simulink model "SunLoadCalcn"  (R2022b)
settings: Solver=FixedStepDiscrete  StartTime=0.0  StopTime=10.0  FixedStep=1  RelTol=1e-3
settings Model: Creator=blue.ge  LastModifiedBy=blue.ge
settings DataIOCC: SaveFormat=StructureWithTime
settings OptimizationCC: DefaultParameterBehavior=Inlined  EnhancedBackFolding=on  PassReuseOutputArgsAs=Individual arguments  ZeroExternalMemoryAtStartup=off  ZeroInternalMemoryAtStartup=off  OptimizeBlockOrder=speed  DifferentSizesBufferReuse=on  OptimizationCustomize=off  EfficientTunableParamExpr=on
settings DebuggingCC: ParameterTunabilityLossMsg=error
settings ModelReferenceCC: ModelReferenceNumInstancesAllowed=Single
settings SFSimCC: MATLABDynamicMemAlloc=off
settings RTWCC: Description=AUTOSAR  SystemTargetFile=autosar.tlc  GenCodeOnly=on  TemplateMakefile=ert_default_tmf
settings CodeAppCC: ForceParamTrailComments=on  IgnoreCustomStorageClasses=off  OperatorAnnotations=on  SimulinkDataObjDesc=on  SFDataObjDesc=on  InsertBlockDesc=on
settings STFCustomTargetCC: TargetLangStandard=C99 (ISO)  MultiwordLength=256  ParMdlRefBuildCompliant=on  CompOptLevelCompliant=on  IncludeMdlTerminateFcn=off  CombineOutputUpdateFcns=on  SuppressErrorStatus=on  ERTCustomFileBanners=on  SupportNonFinite=off  SupportComplex=off  SupportContinuousTime=off  SupportNonInlinedSFcns=off  RemoveResetFunc=on  MATLABClassNameForMDSCustomization=Simulink.SoftwareTarget.ERTCustomization  AutosarCompliant=on  UseToolchainInfoCompliant=on  LookupTableObjectStructAxisOrder=2,1,3,4,...  LUTObjectStructOrderEvenSpacing=Size,Table,Breakpoints  SystemTargetFile=autosar.tlc
settings ERTTargetCC: TargetLangStandard=C99 (ISO)  ConcurrentExecutionCompliant=off  IncludeMdlTerminateFcn=off  SuppressErrorStatus=on  SupportNonFinite=off  SupportComplex=off  ModelStepFunctionPrototypeControlCompliant=off  CPPClassGenCompliant=off  AutosarCompliant=on  LookupTableObjectStructAxisOrder=2,1,3,4,...  LUTObjectStructOrderEvenSpacing=Size,Table,Breakpoints  InlinedParameterPlacement=NonHierarchical  ERTCustomFileTemplate=example_file_process.tlc

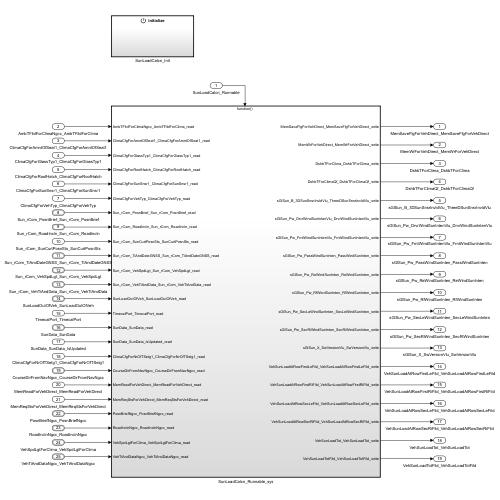
[thumbnail: Simulink's stored preview of the top-level diagram]
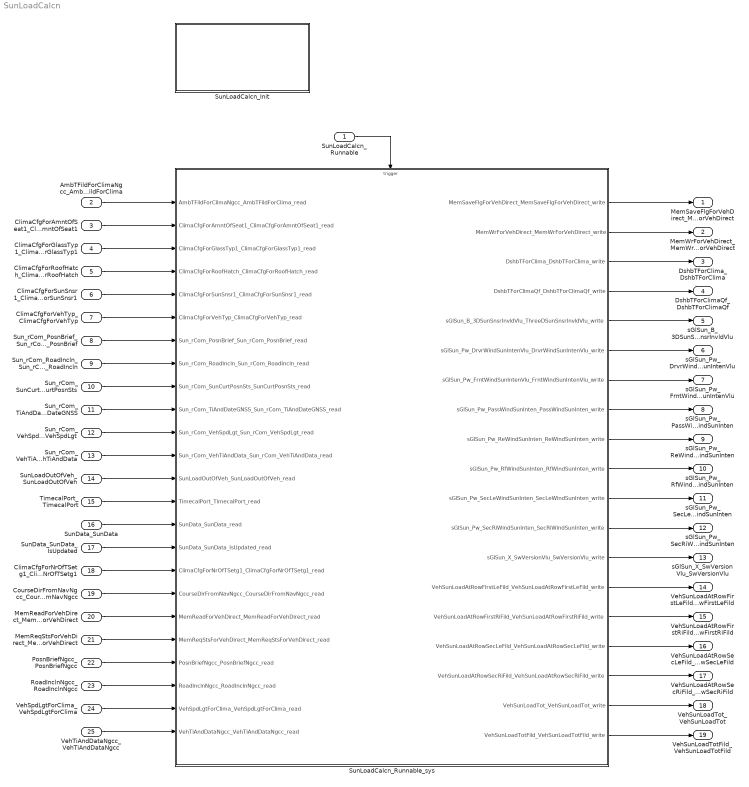
== system SunLoadCalcn ==
  SunLoadCalcn_Runnable : Inport  SampleTime=1  OutputFunctionCall=on
  AmbTFildForClimaNgcc_AmbTFildForClima : Inport  Port=2  OutDataTypeStr=T30  PortDimensions=1
  ClimaCfgForAmntOfSeat1_ClimaCfgForAmntOfSeat1 : Inport  Port=3  OutDataTypeStr=Enum: ClimaCfgForAmntOfSeat1  PortDimensions=1
  ClimaCfgForGlassTyp1_ClimaCfgForGlassTyp1 : Inport  Port=4  OutDataTypeStr=Enum: ClimaCfgForGlassTyp1  PortDimensions=1
  ClimaCfgForRoofHatch_ClimaCfgForRoofHatch : Inport  Port=5  OutDataTypeStr=Enum: CarCfgForRoofHatch  PortDimensions=1
  ClimaCfgForSunSnsr1_ClimaCfgForSunSnsr1 : Inport  Port=6  OutDataTypeStr=Enum: CarCfgForSunSnsr  PortDimensions=1
  ClimaCfgForVehTyp_ClimaCfgForVehTyp : Inport  Port=7  OutDataTypeStr=Enum: CarCfgForVehTyp3  PortDimensions=1
  Sun_rCom_PosnBrief_Sun_rCom_PosnBrief : Inport  Port=8  OutDataTypeStr=Bus: Posn  PortDimensions=1  BusOutputAsStruct=on
  Sun_rCom_RoadIncln_Sun_rCom_RoadIncln : Inport  Port=9  OutDataTypeStr=Bus: RoadInclnRec1  PortDimensions=1  BusOutputAsStruct=on
  Sun_rCom_SunCurtPosnSts_SunCurtPosnSts : Inport  Port=10  OutDataTypeStr=Enum: WinAndRoofAndCurtPosnTyp  PortDimensions=1
  Sun_rCom_TiAndDateGNSS_Sun_rCom_TiAndDateGNSS : Inport  Port=11  OutDataTypeStr=Bus: DateTi30  PortDimensions=1  BusOutputAsStruct=on
  Sun_rCom_VehSpdLgt_Sun_rCom_VehSpdLgt : Inport  Port=12  OutDataTypeStr=Bus: VehSpdLgtSafe1  PortDimensions=1  BusOutputAsStruct=on
  Sun_rCom_VehTiAndData_Sun_rCom_VehTiAndData : Inport  Port=13  OutDataTypeStr=Bus: DateTi30  PortDimensions=1  BusOutputAsStruct=on
  SunLoadOutOfVeh_SunLoadOutOfVeh : Inport  Port=14  OutDataTypeStr=Bus: SunLoadOutOfVeh  PortDimensions=1  BusOutputAsStruct=on
  TimecalPort_TimecalPort : Inport  Port=15  OutDataTypeStr=TimeCal  PortDimensions=1
  SunData_SunData : Inport  Port=16  OutDataTypeStr=Bus: SunData  PortDimensions=1  BusOutputAsStruct=on
  SunData_SunData_IsUpdated : Inport  Port=17  OutDataTypeStr=boolean  PortDimensions=1
  ClimaCfgForNrOfTSetg1_ClimaCfgForNrOfTSetg1 : Inport  Port=18  OutDataTypeStr=Enum: ClimaCfgForNrOfTSetg1  PortDimensions=1
  CourseDirFromNavNgcc_CourseDirFromNavNgcc : Inport  Port=19  OutDataTypeStr=Bus: NavDir  PortDimensions=1  BusOutputAsStruct=on
  MemReadForVehDirect_MemReadForVehDirect : Inport  Port=20  OutDataTypeStr=VCCVehDirect  PortDimensions=1
  MemReqStsForVehDirect_MemReqStsForVehDirect : Inport  Port=21  OutDataTypeStr=Enum: MemReqSts  PortDimensions=1
  PosnBriefNgcc_PosnBriefNgcc : Inport  Port=22  OutDataTypeStr=Bus: Posn  PortDimensions=1  BusOutputAsStruct=on
  RoadInclnNgcc_RoadInclnNgcc : Inport  Port=23  OutDataTypeStr=Bus: RoadInclnRec1  PortDimensions=1  BusOutputAsStruct=on
  VehSpdLgtForClima_VehSpdLgtForClima : Inport  Port=24  OutDataTypeStr=Bus: VehSpdLgtForClima  PortDimensions=1  BusOutputAsStruct=on
  VehTiAndDataNgcc_VehTiAndDataNgcc : Inport  Port=25  OutDataTypeStr=Bus: DateTi30  PortDimensions=1  BusOutputAsStruct=on
  SunLoadCalcn_Init : Subsystem
  SunLoadCalcn_Runnable_sys : Subsystem
  MemSaveFlgForVehDirect_MemSaveFlgForVehDirect : Outport  OutDataTypeStr=Boolean  PortDimensions=1
  MemWrForVehDirect_MemWrForVehDirect : Outport  Port=2  OutDataTypeStr=VCCVehDirect  PortDimensions=1
  DshbTForClima_DshbTForClima : Outport  Port=3  OutDataTypeStr=T30  PortDimensions=1
  DshbTForClimaQf_DshbTForClimaQf : Outport  Port=4  OutDataTypeStr=Enum: DshbTForClimaQf  PortDimensions=1
  sGlSun_B_3DSunSnsrInvldVlu_ThreeDSunSnsrInvldVlu : Outport  Port=5  OutDataTypeStr=Boolean  PortDimensions=1
  sGlSun_Pw_DrvrWindSunIntenVlu_DrvrWindSunIntenVlu : Outport  Port=6  OutDataTypeStr=SunPwr  PortDimensions=1
  sGlSun_Pw_FrntWindSunIntenVlu_FrntWindSunIntenVlu : Outport  Port=7  OutDataTypeStr=SunPwr  PortDimensions=1
  sGlSun_Pw_PassWindSunInten_PassWindSunInten : Outport  Port=8  OutDataTypeStr=SunPwr  PortDimensions=1
  sGlSun_Pw_ReWindSunInten_ReWindSunInten : Outport  Port=9  OutDataTypeStr=SunPwr  PortDimensions=1
  sGlSun_Pw_RfWindSunInten_RfWindSunInten : Outport  Port=10  OutDataTypeStr=SunPwr  PortDimensions=1
  sGlSun_Pw_SecLeWindSunInten_SecLeWindSunInten : Outport  Port=11  OutDataTypeStr=SunPwr  PortDimensions=1
  sGlSun_Pw_SecRiWindSunInten_SecRiWindSunInten : Outport  Port=12  OutDataTypeStr=SunPwr  PortDimensions=1
  sGlSun_X_SwVersionVlu_SwVersionVlu : Outport  Port=13  OutDataTypeStr=UInt8  PortDimensions=1
  VehSunLoadAtRowFirstLeFild_VehSunLoadAtRowFirstLeFild : Outport  Port=14  OutDataTypeStr=SunLoad30  PortDimensions=1
  VehSunLoadAtRowFirstRiFild_VehSunLoadAtRowFirstRiFild : Outport  Port=15  OutDataTypeStr=SunLoad30  PortDimensions=1
  VehSunLoadAtRowSecLeFild_VehSunLoadAtRowSecLeFild : Outport  Port=16  OutDataTypeStr=SunLoad30  PortDimensions=1
  VehSunLoadAtRowSecRiFild_VehSunLoadAtRowSecRiFild : Outport  Port=17  OutDataTypeStr=SunLoad30  PortDimensions=1
  VehSunLoadTot_VehSunLoadTot : Outport  Port=18  OutDataTypeStr=SunLoad30  PortDimensions=1
  VehSunLoadTotFild_VehSunLoadTotFild : Outport  Port=19  OutDataTypeStr=SunLoad30  PortDimensions=1
  wires:
  AmbTFildForClimaNgcc_AmbTFildForClima/1 -> SunLoadCalcn_Runnable_sys/1(AmbTFildForClimaNgcc_AmbTFildForClima_read)
  ClimaCfgForAmntOfSeat1_ClimaCfgForAmntOfSeat1/1 -> SunLoadCalcn_Runnable_sys/2(ClimaCfgForAmntOfSeat1_ClimaCfgForAmntOfSeat1_read)
  ClimaCfgForGlassTyp1_ClimaCfgForGlassTyp1/1 -> SunLoadCalcn_Runnable_sys/3(ClimaCfgForGlassTyp1_ClimaCfgForGlassTyp1_read)
  ClimaCfgForRoofHatch_ClimaCfgForRoofHatch/1 -> SunLoadCalcn_Runnable_sys/4(ClimaCfgForRoofHatch_ClimaCfgForRoofHatch_read)
  ClimaCfgForSunSnsr1_ClimaCfgForSunSnsr1/1 -> SunLoadCalcn_Runnable_sys/5(ClimaCfgForSunSnsr1_ClimaCfgForSunSnsr1_read)
  ClimaCfgForVehTyp_ClimaCfgForVehTyp/1 -> SunLoadCalcn_Runnable_sys/6(ClimaCfgForVehTyp_ClimaCfgForVehTyp_read)
  Sun_rCom_PosnBrief_Sun_rCom_PosnBrief/1 -> SunLoadCalcn_Runnable_sys/7(Sun_rCom_PosnBrief_Sun_rCom_PosnBrief_read)
  Sun_rCom_RoadIncln_Sun_rCom_RoadIncln/1 -> SunLoadCalcn_Runnable_sys/8(Sun_rCom_RoadIncln_Sun_rCom_RoadIncln_read)
  Sun_rCom_SunCurtPosnSts_SunCurtPosnSts/1 -> SunLoadCalcn_Runnable_sys/9(Sun_rCom_SunCurtPosnSts_SunCurtPosnSts_read)
  Sun_rCom_TiAndDateGNSS_Sun_rCom_TiAndDateGNSS/1 -> SunLoadCalcn_Runnable_sys/10(Sun_rCom_TiAndDateGNSS_Sun_rCom_TiAndDateGNSS_read)
  Sun_rCom_VehSpdLgt_Sun_rCom_VehSpdLgt/1 -> SunLoadCalcn_Runnable_sys/11(Sun_rCom_VehSpdLgt_Sun_rCom_VehSpdLgt_read)
  Sun_rCom_VehTiAndData_Sun_rCom_VehTiAndData/1 -> SunLoadCalcn_Runnable_sys/12(Sun_rCom_VehTiAndData_Sun_rCom_VehTiAndData_read)
  SunLoadOutOfVeh_SunLoadOutOfVeh/1 -> SunLoadCalcn_Runnable_sys/13(SunLoadOutOfVeh_SunLoadOutOfVeh_read)
  TimecalPort_TimecalPort/1 -> SunLoadCalcn_Runnable_sys/14(TimecalPort_TimecalPort_read)
  SunData_SunData/1 -> SunLoadCalcn_Runnable_sys/15(SunData_SunData_read)
  SunData_SunData_IsUpdated/1 -> SunLoadCalcn_Runnable_sys/16(SunData_SunData_IsUpdated_read)
  ClimaCfgForNrOfTSetg1_ClimaCfgForNrOfTSetg1/1 -> SunLoadCalcn_Runnable_sys/17(ClimaCfgForNrOfTSetg1_ClimaCfgForNrOfTSetg1_read)
  CourseDirFromNavNgcc_CourseDirFromNavNgcc/1 -> SunLoadCalcn_Runnable_sys/18(CourseDirFromNavNgcc_CourseDirFromNavNgcc_read)
  MemReadForVehDirect_MemReadForVehDirect/1 -> SunLoadCalcn_Runnable_sys/19(MemReadForVehDirect_MemReadForVehDirect_read)
  MemReqStsForVehDirect_MemReqStsForVehDirect/1 -> SunLoadCalcn_Runnable_sys/20(MemReqStsForVehDirect_MemReqStsForVehDirect_read)
  PosnBriefNgcc_PosnBriefNgcc/1 -> SunLoadCalcn_Runnable_sys/21(PosnBriefNgcc_PosnBriefNgcc_read)
  RoadInclnNgcc_RoadInclnNgcc/1 -> SunLoadCalcn_Runnable_sys/22(RoadInclnNgcc_RoadInclnNgcc_read)
  VehSpdLgtForClima_VehSpdLgtForClima/1 -> SunLoadCalcn_Runnable_sys/23(VehSpdLgtForClima_VehSpdLgtForClima_read)
  VehTiAndDataNgcc_VehTiAndDataNgcc/1 -> SunLoadCalcn_Runnable_sys/24(VehTiAndDataNgcc_VehTiAndDataNgcc_read)
  SunLoadCalcn_Runnable_sys/1(MemSaveFlgForVehDirect_MemSaveFlgForVehDirect_write) -> MemSaveFlgForVehDirect_MemSaveFlgForVehDirect/1
  SunLoadCalcn_Runnable_sys/2(MemWrForVehDirect_MemWrForVehDirect_write) -> MemWrForVehDirect_MemWrForVehDirect/1
  SunLoadCalcn_Runnable_sys/3(DshbTForClima_DshbTForClima_write) -> DshbTForClima_DshbTForClima/1
  SunLoadCalcn_Runnable_sys/4(DshbTForClimaQf_DshbTForClimaQf_write) -> DshbTForClimaQf_DshbTForClimaQf/1
  SunLoadCalcn_Runnable_sys/5(sGlSun_B_3DSunSnsrInvldVlu_ThreeDSunSnsrInvldVlu_write) -> sGlSun_B_3DSunSnsrInvldVlu_ThreeDSunSnsrInvldVlu/1
  SunLoadCalcn_Runnable_sys/6(sGlSun_Pw_DrvrWindSunIntenVlu_DrvrWindSunIntenVlu_write) -> sGlSun_Pw_DrvrWindSunIntenVlu_DrvrWindSunIntenVlu/1
  SunLoadCalcn_Runnable_sys/7(sGlSun_Pw_FrntWindSunIntenVlu_FrntWindSunIntenVlu_write) -> sGlSun_Pw_FrntWindSunIntenVlu_FrntWindSunIntenVlu/1
  SunLoadCalcn_Runnable_sys/8(sGlSun_Pw_PassWindSunInten_PassWindSunInten_write) -> sGlSun_Pw_PassWindSunInten_PassWindSunInten/1
  SunLoadCalcn_Runnable_sys/9(sGlSun_Pw_ReWindSunInten_ReWindSunInten_write) -> sGlSun_Pw_ReWindSunInten_ReWindSunInten/1
  SunLoadCalcn_Runnable_sys/10(sGlSun_Pw_RfWindSunInten_RfWindSunInten_write) -> sGlSun_Pw_RfWindSunInten_RfWindSunInten/1
  SunLoadCalcn_Runnable_sys/11(sGlSun_Pw_SecLeWindSunInten_SecLeWindSunInten_write) -> sGlSun_Pw_SecLeWindSunInten_SecLeWindSunInten/1
  SunLoadCalcn_Runnable_sys/12(sGlSun_Pw_SecRiWindSunInten_SecRiWindSunInten_write) -> sGlSun_Pw_SecRiWindSunInten_SecRiWindSunInten/1
  SunLoadCalcn_Runnable_sys/13(sGlSun_X_SwVersionVlu_SwVersionVlu_write) -> sGlSun_X_SwVersionVlu_SwVersionVlu/1
  SunLoadCalcn_Runnable_sys/14(VehSunLoadAtRowFirstLeFild_VehSunLoadAtRowFirstLeFild_write) -> VehSunLoadAtRowFirstLeFild_VehSunLoadAtRowFirstLeFild/1
  SunLoadCalcn_Runnable_sys/15(VehSunLoadAtRowFirstRiFild_VehSunLoadAtRowFirstRiFild_write) -> VehSunLoadAtRowFirstRiFild_VehSunLoadAtRowFirstRiFild/1
  SunLoadCalcn_Runnable_sys/16(VehSunLoadAtRowSecLeFild_VehSunLoadAtRowSecLeFild_write) -> VehSunLoadAtRowSecLeFild_VehSunLoadAtRowSecLeFild/1
  SunLoadCalcn_Runnable_sys/17(VehSunLoadAtRowSecRiFild_VehSunLoadAtRowSecRiFild_write) -> VehSunLoadAtRowSecRiFild_VehSunLoadAtRowSecRiFild/1
  SunLoadCalcn_Runnable_sys/18(VehSunLoadTot_VehSunLoadTot_write) -> VehSunLoadTot_VehSunLoadTot/1
  SunLoadCalcn_Runnable_sys/19(VehSunLoadTotFild_VehSunLoadTotFild_write) -> VehSunLoadTotFild_VehSunLoadTotFild/1
  SunLoadCalcn_Runnable/1 -> SunLoadCalcn_Runnable_sys/Trigger

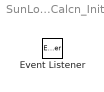
== system SunLoadCalcn/SunLoadCalcn_Init ==
  Event Listener : EventListener  EventName=reset

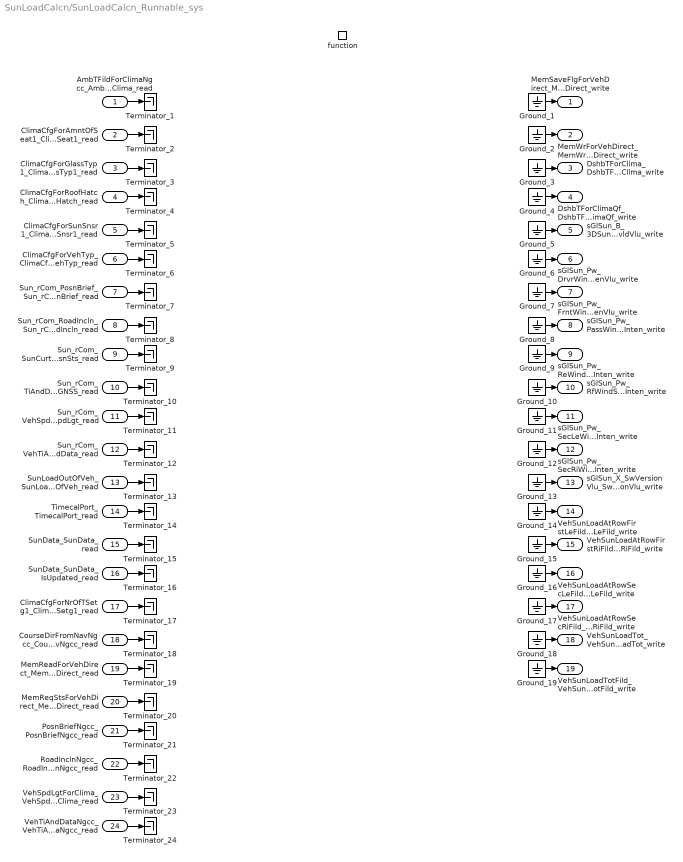
== system SunLoadCalcn/SunLoadCalcn_Runnable_sys ==
  AmbTFildForClimaNgcc_AmbTFildForClima_read : Inport
  ClimaCfgForAmntOfSeat1_ClimaCfgForAmntOfSeat1_read : Inport  Port=2
  ClimaCfgForGlassTyp1_ClimaCfgForGlassTyp1_read : Inport  Port=3
  ClimaCfgForRoofHatch_ClimaCfgForRoofHatch_read : Inport  Port=4
  ClimaCfgForSunSnsr1_ClimaCfgForSunSnsr1_read : Inport  Port=5
  ClimaCfgForVehTyp_ClimaCfgForVehTyp_read : Inport  Port=6
  Sun_rCom_PosnBrief_Sun_rCom_PosnBrief_read : Inport  Port=7
  Sun_rCom_RoadIncln_Sun_rCom_RoadIncln_read : Inport  Port=8
  Sun_rCom_SunCurtPosnSts_SunCurtPosnSts_read : Inport  Port=9
  Sun_rCom_TiAndDateGNSS_Sun_rCom_TiAndDateGNSS_read : Inport  Port=10
  Sun_rCom_VehSpdLgt_Sun_rCom_VehSpdLgt_read : Inport  Port=11
  Sun_rCom_VehTiAndData_Sun_rCom_VehTiAndData_read : Inport  Port=12
  SunLoadOutOfVeh_SunLoadOutOfVeh_read : Inport  Port=13
  TimecalPort_TimecalPort_read : Inport  Port=14
  SunData_SunData_read : Inport  Port=15
  SunData_SunData_IsUpdated_read : Inport  Port=16
  ClimaCfgForNrOfTSetg1_ClimaCfgForNrOfTSetg1_read : Inport  Port=17
  CourseDirFromNavNgcc_CourseDirFromNavNgcc_read : Inport  Port=18
  MemReadForVehDirect_MemReadForVehDirect_read : Inport  Port=19
  MemReqStsForVehDirect_MemReqStsForVehDirect_read : Inport  Port=20
  PosnBriefNgcc_PosnBriefNgcc_read : Inport  Port=21
  RoadInclnNgcc_RoadInclnNgcc_read : Inport  Port=22
  VehSpdLgtForClima_VehSpdLgtForClima_read : Inport  Port=23
  VehTiAndDataNgcc_VehTiAndDataNgcc_read : Inport  Port=24
  function : TriggerPort  TriggerType=function-call  VariantControl=(inherit)  SampleTimeType=periodic  SampleTime=-1
  Ground_{1..19} (19 alike) : Ground
  Terminator_{1..24} (24 alike) : Terminator
  MemSaveFlgForVehDirect_MemSaveFlgForVehDirect_write : Outport
      settings: EnsureOutportIsVirtual=on
  MemWrForVehDirect_MemWrForVehDirect_write : Outport  Port=2
      settings: EnsureOutportIsVirtual=on
  DshbTForClima_DshbTForClima_write : Outport  Port=3
      settings: EnsureOutportIsVirtual=on
  DshbTForClimaQf_DshbTForClimaQf_write : Outport  Port=4
      settings: EnsureOutportIsVirtual=on
  sGlSun_B_3DSunSnsrInvldVlu_ThreeDSunSnsrInvldVlu_write : Outport  Port=5
      settings: EnsureOutportIsVirtual=on
  sGlSun_Pw_DrvrWindSunIntenVlu_DrvrWindSunIntenVlu_write : Outport  Port=6
      settings: EnsureOutportIsVirtual=on
  sGlSun_Pw_FrntWindSunIntenVlu_FrntWindSunIntenVlu_write : Outport  Port=7
      settings: EnsureOutportIsVirtual=on
  sGlSun_Pw_PassWindSunInten_PassWindSunInten_write : Outport  Port=8
      settings: EnsureOutportIsVirtual=on
  sGlSun_Pw_ReWindSunInten_ReWindSunInten_write : Outport  Port=9
      settings: EnsureOutportIsVirtual=on
  sGlSun_Pw_RfWindSunInten_RfWindSunInten_write : Outport  Port=10
      settings: EnsureOutportIsVirtual=on
  sGlSun_Pw_SecLeWindSunInten_SecLeWindSunInten_write : Outport  Port=11
      settings: EnsureOutportIsVirtual=on
  sGlSun_Pw_SecRiWindSunInten_SecRiWindSunInten_write : Outport  Port=12
      settings: EnsureOutportIsVirtual=on
  sGlSun_X_SwVersionVlu_SwVersionVlu_write : Outport  Port=13
      settings: EnsureOutportIsVirtual=on
  VehSunLoadAtRowFirstLeFild_VehSunLoadAtRowFirstLeFild_write : Outport  Port=14
      settings: EnsureOutportIsVirtual=on
  VehSunLoadAtRowFirstRiFild_VehSunLoadAtRowFirstRiFild_write : Outport  Port=15
      settings: EnsureOutportIsVirtual=on
  VehSunLoadAtRowSecLeFild_VehSunLoadAtRowSecLeFild_write : Outport  Port=16
      settings: EnsureOutportIsVirtual=on
  VehSunLoadAtRowSecRiFild_VehSunLoadAtRowSecRiFild_write : Outport  Port=17
      settings: EnsureOutportIsVirtual=on
  VehSunLoadTot_VehSunLoadTot_write : Outport  Port=18
      settings: EnsureOutportIsVirtual=on
  VehSunLoadTotFild_VehSunLoadTotFild_write : Outport  Port=19
      settings: EnsureOutportIsVirtual=on
  wires:
  AmbTFildForClimaNgcc_AmbTFildForClima_read/1 -> Terminator_1/1
  ClimaCfgForAmntOfSeat1_ClimaCfgForAmntOfSeat1_read/1 -> Terminator_2/1
  ClimaCfgForGlassTyp1_ClimaCfgForGlassTyp1_read/1 -> Terminator_3/1
  ClimaCfgForRoofHatch_ClimaCfgForRoofHatch_read/1 -> Terminator_4/1
  ClimaCfgForSunSnsr1_ClimaCfgForSunSnsr1_read/1 -> Terminator_5/1
  ClimaCfgForVehTyp_ClimaCfgForVehTyp_read/1 -> Terminator_6/1
  Sun_rCom_PosnBrief_Sun_rCom_PosnBrief_read/1 -> Terminator_7/1
  Sun_rCom_RoadIncln_Sun_rCom_RoadIncln_read/1 -> Terminator_8/1
  Sun_rCom_SunCurtPosnSts_SunCurtPosnSts_read/1 -> Terminator_9/1
  Sun_rCom_TiAndDateGNSS_Sun_rCom_TiAndDateGNSS_read/1 -> Terminator_10/1
  Sun_rCom_VehSpdLgt_Sun_rCom_VehSpdLgt_read/1 -> Terminator_11/1
  Sun_rCom_VehTiAndData_Sun_rCom_VehTiAndData_read/1 -> Terminator_12/1
  SunLoadOutOfVeh_SunLoadOutOfVeh_read/1 -> Terminator_13/1
  TimecalPort_TimecalPort_read/1 -> Terminator_14/1
  SunData_SunData_read/1 -> Terminator_15/1
  SunData_SunData_IsUpdated_read/1 -> Terminator_16/1
  ClimaCfgForNrOfTSetg1_ClimaCfgForNrOfTSetg1_read/1 -> Terminator_17/1
  CourseDirFromNavNgcc_CourseDirFromNavNgcc_read/1 -> Terminator_18/1
  MemReadForVehDirect_MemReadForVehDirect_read/1 -> Terminator_19/1
  MemReqStsForVehDirect_MemReqStsForVehDirect_read/1 -> Terminator_20/1
  PosnBriefNgcc_PosnBriefNgcc_read/1 -> Terminator_21/1
  RoadInclnNgcc_RoadInclnNgcc_read/1 -> Terminator_22/1
  VehSpdLgtForClima_VehSpdLgtForClima_read/1 -> Terminator_23/1
  VehTiAndDataNgcc_VehTiAndDataNgcc_read/1 -> Terminator_24/1
  Ground_1/1 -> MemSaveFlgForVehDirect_MemSaveFlgForVehDirect_write/1
  Ground_2/1 -> MemWrForVehDirect_MemWrForVehDirect_write/1
  Ground_3/1 -> DshbTForClima_DshbTForClima_write/1
  Ground_4/1 -> DshbTForClimaQf_DshbTForClimaQf_write/1
  Ground_5/1 -> sGlSun_B_3DSunSnsrInvldVlu_ThreeDSunSnsrInvldVlu_write/1
  Ground_6/1 -> sGlSun_Pw_DrvrWindSunIntenVlu_DrvrWindSunIntenVlu_write/1
  Ground_7/1 -> sGlSun_Pw_FrntWindSunIntenVlu_FrntWindSunIntenVlu_write/1
  Ground_8/1 -> sGlSun_Pw_PassWindSunInten_PassWindSunInten_write/1
  Ground_9/1 -> sGlSun_Pw_ReWindSunInten_ReWindSunInten_write/1
  Ground_10/1 -> sGlSun_Pw_RfWindSunInten_RfWindSunInten_write/1
  Ground_11/1 -> sGlSun_Pw_SecLeWindSunInten_SecLeWindSunInten_write/1
  Ground_12/1 -> sGlSun_Pw_SecRiWindSunInten_SecRiWindSunInten_write/1
  Ground_13/1 -> sGlSun_X_SwVersionVlu_SwVersionVlu_write/1
  Ground_14/1 -> VehSunLoadAtRowFirstLeFild_VehSunLoadAtRowFirstLeFild_write/1
  Ground_15/1 -> VehSunLoadAtRowFirstRiFild_VehSunLoadAtRowFirstRiFild_write/1
  Ground_16/1 -> VehSunLoadAtRowSecLeFild_VehSunLoadAtRowSecLeFild_write/1
  Ground_17/1 -> VehSunLoadAtRowSecRiFild_VehSunLoadAtRowSecRiFild_write/1
  Ground_18/1 -> VehSunLoadTot_VehSunLoadTot_write/1
  Ground_19/1 -> VehSunLoadTotFild_VehSunLoadTotFild_write/1
